simulink model "os_polyphase_synth_4096c"  (R2021a) (continued)
== system os_polyphase_synth_4096c/pfb_fir/pol1_in1_tap55 == (continued)
      | -P+(D+A)*BCIN+P
      | -P+(D+A)+C
      | -P+(D+A)+P
      | -P+(D+ACIN)
      | -P+(D+ACIN)*(D+ACIN)
      | -P+(D+ACIN)*(D+ACIN)+C
      | -P+(D+ACIN)*(D+ACIN)+P
      | -P+(D+ACIN)*B
      | -P+(D+ACIN)*B+C
      | -P+(D+ACIN)*B+P
      | -P+(D+ACIN)*BCIN
      | -P+(D+ACIN)*BCIN+C
      | -P+(D+ACIN)*BCIN+P
      | -P+(D+ACIN)+C
      | -P+(D+ACIN)+P
      | -P+(D-A)
      | -P+(D-A)*(D-A)
      | -P+(D-A)*(D-A)+C
      | -P+(D-A)*(D-A)+P
      | -P+(D-A)*B
      | -P+(D-A)*B+C
      | -P+(D-A)*B+P
      | -P+(D-A)*BCIN
      | -P+(D-A)*BCIN+C
      | -P+(D-A)*BCIN+P
      | -P+(D-A)+C
      | -P+(D-A)+P
      | -P+(D-ACIN)
      | -P+(D-ACIN)*(D-ACIN)
      | -P+(D-ACIN)*(D-ACIN)+C
      | -P+(D-ACIN)*(D-ACIN)+P
      | -P+(D-ACIN)*B
      | -P+(D-ACIN)*B+C
      | -P+(D-ACIN)*B+P
      | -P+(D-ACIN)*BCIN
      | -P+(D-ACIN)*BCIN+C
      | -P+(D-ACIN)*BCIN+P
      | -P+(D-ACIN)+C
      | -P+(D-ACIN)+P
      | -P+A
      | -P+A*A
      | -P+A*A+C
      | -P+A*A+P
      | -P+A*B
      | -P+A*B+C
      | -P+A*B+P
      | -P+A*BCIN
      | -P+A*BCIN+C
      | -P+A*BCIN+P
      | -P+A+C
      | -P+A+P
      | -P+ACIN
      | -P+ACIN*ACIN
      | -P+ACIN*ACIN+C
      | -P+ACIN*ACIN+P
      | -P+ACIN*B
      | -P+ACIN*B+C
      | -P+ACIN*B+P
      | -P+ACIN*BCIN
      | -P+ACIN*BCIN+C
      | -P+ACIN*BCIN+P
      | -P+ACIN+C
      | -P+ACIN+P
      | -P+B*(A+D)
      | -P+B*(A+D)+C
      | -P+B*(A+D)+P
      | -P+B*(ACIN+D)
      | -P+B*(ACIN+D)+C
      | -P+B*(ACIN+D)+P
      | -P+B*(D+A)
      | -P+B*(D+A)+C
      | -P+B*(D+A)+P
      | -P+B*(D+ACIN)
      | -P+B*(D+ACIN)+C
      | -P+B*(D+ACIN)+P
      | -P+B*(D-A)
      | -P+B*(D-A)+C
      | -P+B*(D-A)+P
      | -P+B*(D-ACIN)
      | -P+B*(D-ACIN)+C
      | -P+B*(D-ACIN)+P
      | -P+B*A
      | -P+B*A+C
      | -P+B*A+P
      | -P+B*ACIN
      | -P+B*ACIN+C
      | -P+B*ACIN+P
      | -P+B*D
      | -P+B*D+C
      | -P+B*D+P
      | -P+BCIN*(A+D)
      | -P+BCIN*(A+D)+C
      | -P+BCIN*(A+D)+P
      | -P+BCIN*(ACIN+D)
      | -P+BCIN*(ACIN+D)+C
      | -P+BCIN*(ACIN+D)+P
      | -P+BCIN*(D+A)
      | -P+BCIN*(D+A)+C
      | -P+BCIN*(D+A)+P
      | -P+BCIN*(D+ACIN)
      | -P+BCIN*(D+ACIN)+C
      | -P+BCIN*(D+ACIN)+P
      | -P+BCIN*(D-A)
      | -P+BCIN*(D-A)+C
      | -P+BCIN*(D-A)+P
      | -P+BCIN*(D-ACIN)
      | -P+BCIN*(D-ACIN)+C
      | -P+BCIN*(D-ACIN)+P
      | -P+BCIN*A
      | -P+BCIN*A+C
      | -P+BCIN*A+P
      | -P+BCIN*ACIN
      | -P+BCIN*ACIN+C
      | -P+BCIN*ACIN+P
      | -P+BCIN*D
      | -P+BCIN*D+C
      | -P+BCIN*D+P
      | -P+C
      | -P+C+(A+D)
      | -P+C+(A+D)*(A+D)
      | -P+C+(A+D)*B
      | -P+C+(A+D)*BCIN
      | -P+C+(ACIN+D)
      | -P+C+(ACIN+D)*(ACIN+D)
      | -P+C+(ACIN+D)*B
      | -P+C+(ACIN+D)*BCIN
      | -P+C+(D+A)
      | -P+C+(D+A)*(D+A)
      | -P+C+(D+A)*B
      | -P+C+(D+A)*BCIN
      | -P+C+(D+ACIN)
      | -P+C+(D+ACIN)*(D+ACIN)
      | -P+C+(D+ACIN)*B
      | -P+C+(D+ACIN)*BCIN
      | -P+C+(D-A)
      | -P+C+(D-A)*(D-A)
      | -P+C+(D-A)*B
      | -P+C+(D-A)*BCIN
      | -P+C+(D-ACIN)
      | -P+C+(D-ACIN)*(D-ACIN)
      | -P+C+(D-ACIN)*B
      | -P+C+(D-ACIN)*BCIN
      | -P+C+A
      | -P+C+A*A
      | -P+C+A*B
      | -P+C+A*BCIN
      | -P+C+ACIN
      | -P+C+ACIN*ACIN
      | -P+C+ACIN*B
      | -P+C+ACIN*BCIN
      | -P+C+B*(A+D)
      | -P+C+B*(ACIN+D)
      | -P+C+B*(D+A)
      | -P+C+B*(D+ACIN)
      | -P+C+B*(D-A)
      | -P+C+B*(D-ACIN)
      | -P+C+B*A
      | -P+C+B*ACIN
      | -P+C+B*D
      | -P+C+BCIN*(A+D)
      | -P+C+BCIN*(ACIN+D)
      | -P+C+BCIN*(D+A)
      | -P+C+BCIN*(D+ACIN)
      | -P+C+BCIN*(D-A)
      | -P+C+BCIN*(D-ACIN)
      | -P+C+BCIN*A
      | -P+C+BCIN*ACIN
      | -P+C+BCIN*D
      | -P+C+C
      | -P+C+C+CONCAT
      | -P+C+C+P
      | -P+C+CONCAT
      | -P+C+CONCAT+C
      | -P+C+CONCAT+P
      | -P+C+D
      | -P+C+D*B
      | -P+C+D*BCIN
      | -P+C+D*D
      | -P+C+P
      | -P+C+P+C
      | -P+C+P+CONCAT
      | -P+C+P+P
      | -P+CONCAT
      | -P+CONCAT+C
      | -P+CONCAT+C+C
      | -P+CONCAT+C+P
      | -P+CONCAT+P
      | -P+CONCAT+P+C
      | -P+D
      | -P+D*B
      | -P+D*B+C
      | -P+D*B+P
      | -P+D*BCIN
      | -P+D*BCIN+C
      | -P+D*BCIN+P
      | -P+D*D
      | -P+D*D+C
      | -P+D*D+P
      | -P+D+C
      | -P+D+P
      | -P+P
      | -P+P+(A+D)
      | -P+P+(A+D)*(A+D)
      | -P+P+(A+D)*B
      | -P+P+(A+D)*BCIN
      | -P+P+(ACIN+D)
      | -P+P+(ACIN+D)*(ACIN+D)
      | -P+P+(ACIN+D)*B
      | -P+P+(ACIN+D)*BCIN
      | -P+P+(D+A)
      | -P+P+(D+A)*(D+A)
      | -P+P+(D+A)*B
      | -P+P+(D+A)*BCIN
      | -P+P+(D+ACIN)
      | -P+P+(D+ACIN)*(D+ACIN)
      | -P+P+(D+ACIN)*B
      | -P+P+(D+ACIN)*BCIN
      | -P+P+(D-A)
      | -P+P+(D-A)*(D-A)
      | -P+P+(D-A)*B
      | -P+P+(D-A)*BCIN
      | -P+P+(D-ACIN)
      | -P+P+(D-ACIN)*(D-ACIN)
      | -P+P+(D-ACIN)*B
      | -P+P+(D-ACIN)*BCIN
      | -P+P+A
      | -P+P+A*A
      | -P+P+A*B
      | -P+P+A*BCIN
      | -P+P+ACIN
      | -P+P+ACIN*ACIN
      | -P+P+ACIN*B
      | -P+P+ACIN*BCIN
      | -P+P+B*(A+D)
      | -P+P+B*(ACIN+D)
      | -P+P+B*(D+A)
      | -P+P+B*(D+ACIN)
      | -P+P+B*(D-A)
      | -P+P+B*(D-ACIN)
      | -P+P+B*A
      | -P+P+B*ACIN
      | -P+P+B*D
      | -P+P+BCIN*(A+D)
      | -P+P+BCIN*(ACIN+D)
      | -P+P+BCIN*(D+A)
      | -P+P+BCIN*(D+ACIN)
      | -P+P+BCIN*(D-A)
      | -P+P+BCIN*(D-ACIN)
      | -P+P+BCIN*A
      | -P+P+BCIN*ACIN
      | -P+P+BCIN*D
      | -P+P+C
      | -P+P+C+C
      | -P+P+C+CONCAT
      | -P+P+C+P
      | -P+P+CONCAT
      | -P+P+CONCAT+C
      | -P+P+D
      | -P+P+D*B
      | -P+P+D*BCIN
      | -P+P+D*D
      | -P+P+P
      | -P+P+P+C
      | -P-((A+D))
      | -P-((A+D)*(A+D))
      | -P-((A+D)*B)
      | -P-((A+D)*BCIN)
      | -P-((ACIN+D))
      | -P-((ACIN+D)*(ACIN+D))
      | -P-((ACIN+D)*B)
      | -P-((ACIN+D)*BCIN)
      | -P-((D+A))
      | -P-((D+A)*(D+A))
      | -P-((D+A)*B)
      | -P-((D+A)*BCIN)
      | -P-((D+ACIN))
      | -P-((D+ACIN)*(D+ACIN))
      | -P-((D+ACIN)*B)
      | -P-((D+ACIN)*BCIN)
      | -P-((D-A))
      | -P-((D-A)*(D-A))
      | -P-((D-A)*B)
      | -P-((D-A)*BCIN)
      | -P-((D-ACIN))
      | -P-((D-ACIN)*(D-ACIN))
      | -P-((D-ACIN)*B)
      | -P-((D-ACIN)*BCIN)
      | -P-(A)
      | -P-(A*A)
      | -P-(A*B)
      | -P-(A*BCIN)
      | -P-(A+D)
      | -P-(A+D)*(A+D)
      | -P-(A+D)*(A+D)-CARRYIN
      | -P-(A+D)*B
      | -P-(A+D)*B-CARRYIN
      | -P-(A+D)*BCIN
      | -P-(A+D)*BCIN-CARRYIN
      | -P-(A+D)-CARRYIN
      | -P-(ACIN)
      | -P-(ACIN*ACIN)
      | -P-(ACIN*B)
      | -P-(ACIN*BCIN)
      | -P-(ACIN+D)
      | -P-(ACIN+D)*(ACIN+D)
      | -P-(ACIN+D)*(ACIN+D)-CARRYIN
      | -P-(ACIN+D)*B
      | -P-(ACIN+D)*B-CARRYIN
      | -P-(ACIN+D)*BCIN
      | -P-(ACIN+D)*BCIN-CARRYIN
      | -P-(ACIN+D)-CARRYIN
      | -P-(B*(A+D))
      | -P-(B*(ACIN+D))
      | -P-(B*(D+A))
      | -P-(B*(D+ACIN))
      | -P-(B*(D-A))
      | -P-(B*(D-ACIN))
      | -P-(B*A)
      | -P-(B*ACIN)
      | -P-(B*D)
      | -P-(BCIN*(A+D))
      | -P-(BCIN*(ACIN+D))
      | -P-(BCIN*(D+A))
      | -P-(BCIN*(D+ACIN))
      | -P-(BCIN*(D-A))
      | -P-(BCIN*(D-ACIN))
      | -P-(BCIN*A)
      | -P-(BCIN*ACIN)
      | -P-(BCIN*D)
      | -P-(D)
      | -P-(D*B)
      | -P-(D*BCIN)
      | -P-(D*D)
      | -P-(D+A)
      | -P-(D+A)*(D+A)
      | -P-(D+A)*(D+A)-CARRYIN
      | -P-(D+A)*B
      | -P-(D+A)*B-CARRYIN
      | -P-(D+A)*BCIN
      | -P-(D+A)*BCIN-CARRYIN
      | -P-(D+A)-CARRYIN
      | -P-(D+ACIN)
      | -P-(D+ACIN)*(D+ACIN)
      | -P-(D+ACIN)*(D+ACIN)-CARRYIN
      | -P-(D+ACIN)*B
      | -P-(D+ACIN)*B-CARRYIN
      | -P-(D+ACIN)*BCIN
      | -P-(D+ACIN)*BCIN-CARRYIN
      | -P-(D+ACIN)-CARRYIN
      | -P-(D-A)
      | -P-(D-A)*(D-A)
      | -P-(D-A)*(D-A)-CARRYIN
      | -P-(D-A)*B
      | -P-(D-A)*B-CARRYIN
      | -P-(D-A)*BCIN
      | -P-(D-A)*BCIN-CARRYIN
      | -P-(D-A)-CARRYIN
      | -P-(D-ACIN)
      | -P-(D-ACIN)*(D-ACIN)
      | -P-(D-ACIN)*(D-ACIN)-CARRYIN
      | -P-(D-ACIN)*B
      | -P-(D-ACIN)*B-CARRYIN
      | -P-(D-ACIN)*BCIN
      | -P-(D-ACIN)*BCIN-CARRYIN
      | -P-(D-ACIN)-CARRYIN
      | -P-A
      | -P-A*A
      | -P-A*A-CARRYIN
      | -P-A*B
      | -P-A*B-CARRYIN
      | -P-A*BCIN
      | -P-A*BCIN-CARRYIN
      | -P-A-CARRYIN
      | -P-ACIN
      | -P-ACIN*ACIN
      | -P-ACIN*ACIN-CARRYIN
      | -P-ACIN*B
      | -P-ACIN*B-CARRYIN
      | -P-ACIN*BCIN
      | -P-ACIN*BCIN-CARRYIN
      | -P-ACIN-CARRYIN
      | -P-B*(A+D)
      | -P-B*(A+D)-CARRYIN
      | -P-B*(ACIN+D)
      | -P-B*(ACIN+D)-CARRYIN
      | -P-B*(D+A)
      | -P-B*(D+A)-CARRYIN
      | -P-B*(D+ACIN)
      | -P-B*(D+ACIN)-CARRYIN
      | -P-B*(D-A)
      | -P-B*(D-A)-CARRYIN
      | -P-B*(D-ACIN)
      | -P-B*(D-ACIN)-CARRYIN
      | -P-B*A
      | -P-B*A-CARRYIN
      | -P-B*ACIN
      | -P-B*ACIN-CARRYIN
      | -P-B*D
      | -P-B*D-CARRYIN
      | -P-BCIN*(A+D)
      | -P-BCIN*(A+D)-CARRYIN
      | -P-BCIN*(ACIN+D)
      | -P-BCIN*(ACIN+D)-CARRYIN
      | -P-BCIN*(D+A)
      | -P-BCIN*(D+A)-CARRYIN
      | -P-BCIN*(D+ACIN)
      | -P-BCIN*(D+ACIN)-CARRYIN
      | -P-BCIN*(D-A)
      | -P-BCIN*(D-A)-CARRYIN
      | -P-BCIN*(D-ACIN)
      | -P-BCIN*(D-ACIN)-CARRYIN
      | -P-BCIN*A
      | -P-BCIN*A-CARRYIN
      | -P-BCIN*ACIN
      | -P-BCIN*ACIN-CARRYIN
      | -P-BCIN*D
      | -P-BCIN*D-CARRYIN
      | -P-C
      | -P-C-C
      | -P-C-C-CARRYIN
      | -P-C-CARRYCASCIN
      | -P-C-CARRYIN
      | -P-C-CONCAT
      | -P-C-CONCAT-CARRYIN
      | -P-C-P
      | -P-C-P-CARRYIN
      | -P-C-P>>17
      | -P-C-PCIN
      | -P-C-PCIN>>17
      | -P-CARRYCASCIN
      | -P-CARRYIN
      | -P-CONCAT
      | -P-CONCAT-C
      | -P-CONCAT-C-CARRYIN
      | -P-CONCAT-CARRYCASCIN
      | -P-CONCAT-CARRYIN
      | -P-CONCAT-P
      | -P-CONCAT-P>>17
      | -P-CONCAT-PCIN
      | -P-CONCAT-PCIN>>17
      | -P-D
      | -P-D*B
      | -P-D*B-CARRYIN
      | -P-D*BCIN
      | -P-D*BCIN-CARRYIN
      | -P-D*D
      | -P-D*D-CARRYIN
      | -P-D-CARRYIN
      | -P-P
      | -P-P-C
      | -P-P-C-CARRYIN
      | -P-P-CARRYCASCIN
      | -P-P-CARRYIN
      | -P-P-P
      | -P-P-P>>17
      | -P-P-PCIN
      | -P-P-PCIN>>17
      | -P-P>>17
      | -P-PCIN
      | -P-PCIN>>17
      | -P>>17
      | -P>>17+(A+D)
      | -P>>17+(A+D)*(A+D)
      | -P>>17+(A+D)*(A+D)+C
      | -P>>17+(A+D)*(A+D)+P
      | -P>>17+(A+D)*B
      | -P>>17+(A+D)*B+C
      | -P>>17+(A+D)*B+P
      | -P>>17+(A+D)*BCIN
      | -P>>17+(A+D)*BCIN+C
      | -P>>17+(A+D)*BCIN+P
      | -P>>17+(A+D)+C
      | -P>>17+(A+D)+P
      | -P>>17+(ACIN+D)
      | -P>>17+(ACIN+D)*(ACIN+D)
      | -P>>17+(ACIN+D)*(ACIN+D)+C
      | -P>>17+(ACIN+D)*(ACIN+D)+P
      | -P>>17+(ACIN+D)*B
      | -P>>17+(ACIN+D)*B+C
      | -P>>17+(ACIN+D)*B+P
      | -P>>17+(ACIN+D)*BCIN
      | -P>>17+(ACIN+D)*BCIN+C
      | -P>>17+(ACIN+D)*BCIN+P
      | -P>>17+(ACIN+D)+C
      | -P>>17+(ACIN+D)+P
      | -P>>17+(D+A)
      | -P>>17+(D+A)*(D+A)
      | -P>>17+(D+A)*(D+A)+C
      | -P>>17+(D+A)*(D+A)+P
      | -P>>17+(D+A)*B
      | -P>>17+(D+A)*B+C
      | -P>>17+(D+A)*B+P
      | -P>>17+(D+A)*BCIN
      | -P>>17+(D+A)*BCIN+C
      | -P>>17+(D+A)*BCIN+P
      | -P>>17+(D+A)+C
      | -P>>17+(D+A)+P
      | -P>>17+(D+ACIN)
      | -P>>17+(D+ACIN)*(D+ACIN)
      | -P>>17+(D+ACIN)*(D+ACIN)+C
      | -P>>17+(D+ACIN)*(D+ACIN)+P
      | -P>>17+(D+ACIN)*B
      | -P>>17+(D+ACIN)*B+C
      | -P>>17+(D+ACIN)*B+P
      | -P>>17+(D+ACIN)*BCIN
      | -P>>17+(D+ACIN)*BCIN+C
      | -P>>17+(D+ACIN)*BCIN+P
      | -P>>17+(D+ACIN)+C
      | -P>>17+(D+ACIN)+P
      | -P>>17+(D-A)
      | -P>>17+(D-A)*(D-A)
      | -P>>17+(D-A)*(D-A)+C
      | -P>>17+(D-A)*(D-A)+P
      | -P>>17+(D-A)*B
      | -P>>17+(D-A)*B+C
      | -P>>17+(D-A)*B+P
      | -P>>17+(D-A)*BCIN
      | -P>>17+(D-A)*BCIN+C
      | -P>>17+(D-A)*BCIN+P
      | -P>>17+(D-A)+C
      | -P>>17+(D-A)+P
      | -P>>17+(D-ACIN)
      | -P>>17+(D-ACIN)*(D-ACIN)
      | -P>>17+(D-ACIN)*(D-ACIN)+C
      | -P>>17+(D-ACIN)*(D-ACIN)+P
      | -P>>17+(D-ACIN)*B
      | -P>>17+(D-ACIN)*B+C
      | -P>>17+(D-ACIN)*B+P
      | -P>>17+(D-ACIN)*BCIN
      | -P>>17+(D-ACIN)*BCIN+C
      | -P>>17+(D-ACIN)*BCIN+P
      | -P>>17+(D-ACIN)+C
      | -P>>17+(D-ACIN)+P
      | -P>>17+A
      | -P>>17+A*A
      | -P>>17+A*A+C
      | -P>>17+A*A+P
      | -P>>17+A*B
      | -P>>17+A*B+C
      | -P>>17+A*B+P
      | -P>>17+A*BCIN
      | -P>>17+A*BCIN+C
      | -P>>17+A*BCIN+P
      | -P>>17+A+C
      | -P>>17+A+P
      | -P>>17+ACIN
      | -P>>17+ACIN*ACIN
      | -P>>17+ACIN*ACIN+C
      | -P>>17+ACIN*ACIN+P
      | -P>>17+ACIN*B
      | -P>>17+ACIN*B+C
      | -P>>17+ACIN*B+P
      | -P>>17+ACIN*BCIN
      | -P>>17+ACIN*BCIN+C
      | -P>>17+ACIN*BCIN+P
      | -P>>17+ACIN+C
      | -P>>17+ACIN+P
      | -P>>17+B*(A+D)
      | -P>>17+B*(A+D)+C
      | -P>>17+B*(A+D)+P
      | -P>>17+B*(ACIN+D)
      | -P>>17+B*(ACIN+D)+C
      | -P>>17+B*(ACIN+D)+P
      | -P>>17+B*(D+A)
      | -P>>17+B*(D+A)+C
      | -P>>17+B*(D+A)+P
      | -P>>17+B*(D+ACIN)
      | -P>>17+B*(D+ACIN)+C
      | -P>>17+B*(D+ACIN)+P
      | -P>>17+B*(D-A)
      | -P>>17+B*(D-A)+C
      | -P>>17+B*(D-A)+P
      | -P>>17+B*(D-ACIN)
      | -P>>17+B*(D-ACIN)+C
      | -P>>17+B*(D-ACIN)+P
      | -P>>17+B*A
      | -P>>17+B*A+C
      | -P>>17+B*A+P
      | -P>>17+B*ACIN
      | -P>>17+B*ACIN+C
      | -P>>17+B*ACIN+P
      | -P>>17+B*D
      | -P>>17+B*D+C
      | -P>>17+B*D+P
      | -P>>17+BCIN*(A+D)
      | -P>>17+BCIN*(A+D)+C
      | -P>>17+BCIN*(A+D)+P
      | -P>>17+BCIN*(ACIN+D)
      | -P>>17+BCIN*(ACIN+D)+C
      | -P>>17+BCIN*(ACIN+D)+P
      | -P>>17+BCIN*(D+A)
      | -P>>17+BCIN*(D+A)+C
      | -P>>17+BCIN*(D+A)+P
      | -P>>17+BCIN*(D+ACIN)
      | -P>>17+BCIN*(D+ACIN)+C
      | -P>>17+BCIN*(D+ACIN)+P
      | -P>>17+BCIN*(D-A)
      | -P>>17+BCIN*(D-A)+C
      | -P>>17+BCIN*(D-A)+P
      | -P>>17+BCIN*(D-ACIN)
      | -P>>17+BCIN*(D-ACIN)+C
      | -P>>17+BCIN*(D-ACIN)+P
      | -P>>17+BCIN*A
      | -P>>17+BCIN*A+C
      | -P>>17+BCIN*A+P
      | -P>>17+BCIN*ACIN
      | -P>>17+BCIN*ACIN+C
      | -P>>17+BCIN*ACIN+P
      | -P>>17+BCIN*D
      | -P>>17+BCIN*D+C
      | -P>>17+BCIN*D+P
      | -P>>17+C
      | -P>>17+C+(A+D)
      | -P>>17+C+(A+D)*(A+D)
      | -P>>17+C+(A+D)*B
      | -P>>17+C+(A+D)*BCIN
      | -P>>17+C+(ACIN+D)
      | -P>>17+C+(ACIN+D)*(ACIN+D)
      | -P>>17+C+(ACIN+D)*B
      | -P>>17+C+(ACIN+D)*BCIN
      | -P>>17+C+(D+A)
      | -P>>17+C+(D+A)*(D+A)
      | -P>>17+C+(D+A)*B
      | -P>>17+C+(D+A)*BCIN
      | -P>>17+C+(D+ACIN)
      | -P>>17+C+(D+ACIN)*(D+ACIN)
      | -P>>17+C+(D+ACIN)*B
      | -P>>17+C+(D+ACIN)*BCIN
      | -P>>17+C+(D-A)
      | -P>>17+C+(D-A)*(D-A)
      | -P>>17+C+(D-A)*B
      | -P>>17+C+(D-A)*BCIN
      | -P>>17+C+(D-ACIN)
      | -P>>17+C+(D-ACIN)*(D-ACIN)
      | -P>>17+C+(D-ACIN)*B
      | -P>>17+C+(D-ACIN)*BCIN
      | -P>>17+C+A
      | -P>>17+C+A*A
      | -P>>17+C+A*B
      | -P>>17+C+A*BCIN
      | -P>>17+C+ACIN
      | -P>>17+C+ACIN*ACIN
      | -P>>17+C+ACIN*B
      | -P>>17+C+ACIN*BCIN
      | -P>>17+C+B*(A+D)
      | -P>>17+C+B*(ACIN+D)
      | -P>>17+C+B*(D+A)
      | -P>>17+C+B*(D+ACIN)
      | -P>>17+C+B*(D-A)
      | -P>>17+C+B*(D-ACIN)
      | -P>>17+C+B*A
      | -P>>17+C+B*ACIN
      | -P>>17+C+B*D
      | -P>>17+C+BCIN*(A+D)
      | -P>>17+C+BCIN*(ACIN+D)
      | -P>>17+C+BCIN*(D+A)
      | -P>>17+C+BCIN*(D+ACIN)
      | -P>>17+C+BCIN*(D-A)
      | -P>>17+C+BCIN*(D-ACIN)
      | -P>>17+C+BCIN*A
      | -P>>17+C+BCIN*ACIN
      | -P>>17+C+BCIN*D
      | -P>>17+C+C
      | -P>>17+C+C+CONCAT
      | -P>>17+C+C+P
      | -P>>17+C+CONCAT
      | -P>>17+C+CONCAT+C
      | -P>>17+C+CONCAT+P
      | -P>>17+C+D
      | -P>>17+C+D*B
      | -P>>17+C+D*BCIN
      | -P>>17+C+D*D
      | -P>>17+C+P
      | -P>>17+C+P+C
      | -P>>17+C+P+CONCAT
      | -P>>17+C+P+P
      | -P>>17+CONCAT
      | -P>>17+CONCAT+C
      | -P>>17+CONCAT+C+C
      | -P>>17+CONCAT+C+P
      | -P>>17+CONCAT+P
      | -P>>17+CONCAT+P+C
      | -P>>17+D
      | -P>>17+D*B
      | -P>>17+D*B+C
      | -P>>17+D*B+P
      | -P>>17+D*BCIN
      | -P>>17+D*BCIN+C
      | -P>>17+D*BCIN+P
      | -P>>17+D*D
      | -P>>17+D*D+C
      | -P>>17+D*D+P
      | -P>>17+D+C
      | -P>>17+D+P
      | -P>>17+P
      | -P>>17+P+(A+D)
      | -P>>17+P+(A+D)*(A+D)
      | -P>>17+P+(A+D)*B
      | -P>>17+P+(A+D)*BCIN
      | -P>>17+P+(ACIN+D)
      | -P>>17+P+(ACIN+D)*(ACIN+D)
      | -P>>17+P+(ACIN+D)*B
      | -P>>17+P+(ACIN+D)*BCIN
      | -P>>17+P+(D+A)
      | -P>>17+P+(D+A)*(D+A)
      | -P>>17+P+(D+A)*B
      | -P>>17+P+(D+A)*BCIN
      | -P>>17+P+(D+ACIN)
      | -P>>17+P+(D+ACIN)*(D+ACIN)
      | -P>>17+P+(D+ACIN)*B
      | -P>>17+P+(D+ACIN)*BCIN
      | -P>>17+P+(D-A)
      | -P>>17+P+(D-A)*(D-A)
      | -P>>17+P+(D-A)*B
      | -P>>17+P+(D-A)*BCIN
      | -P>>17+P+(D-ACIN)
      | -P>>17+P+(D-ACIN)*(D-ACIN)
      | -P>>17+P+(D-ACIN)*B
      | -P>>17+P+(D-ACIN)*BCIN
      | -P>>17+P+A
      | -P>>17+P+A*A
      | -P>>17+P+A*B
      | -P>>17+P+A*BCIN
      | -P>>17+P+ACIN
      | -P>>17+P+ACIN*ACIN
      | -P>>17+P+ACIN*B
      | -P>>17+P+ACIN*BCIN
      | -P>>17+P+B*(A+D)
      | -P>>17+P+B*(ACIN+D)
      | -P>>17+P+B*(D+A)
      | -P>>17+P+B*(D+ACIN)
      | -P>>17+P+B*(D-A)
      | -P>>17+P+B*(D-ACIN)
      | -P>>17+P+B*A
      | -P>>17+P+B*ACIN
      | -P>>17+P+B*D
      | -P>>17+P+BCIN*(A+D)
      | -P>>17+P+BCIN*(ACIN+D)
      | -P>>17+P+BCIN*(D+A)
      | -P>>17+P+BCIN*(D+ACIN)
      | -P>>17+P+BCIN*(D-A)
      | -P>>17+P+BCIN*(D-ACIN)
      | -P>>17+P+BCIN*A
      | -P>>17+P+BCIN*ACIN
      | -P>>17+P+BCIN*D
      | -P>>17+P+C
      | -P>>17+P+C+C
      | -P>>17+P+C+CONCAT
      | -P>>17+P+C+P
      | -P>>17+P+CONCAT
      | -P>>17+P+CONCAT+C
      | -P>>17+P+D
      | -P>>17+P+D*B
      | -P>>17+P+D*BCIN
      | -P>>17+P+D*D
      | -P>>17+P+P
      | -P>>17+P+P+C
      | -P>>17-((A+D))
      | -P>>17-((A+D)*(A+D))
      | -P>>17-((A+D)*B)
      | -P>>17-((A+D)*BCIN)
      | -P>>17-((ACIN+D))
      | -P>>17-((ACIN+D)*(ACIN+D))
      | -P>>17-((ACIN+D)*B)
      | -P>>17-((ACIN+D)*BCIN)
      | -P>>17-((D+A))
      | -P>>17-((D+A)*(D+A))
      | -P>>17-((D+A)*B)
      | -P>>17-((D+A)*BCIN)
      | -P>>17-((D+ACIN))
      | -P>>17-((D+ACIN)*(D+ACIN))
      | -P>>17-((D+ACIN)*B)
      | -P>>17-((D+ACIN)*BCIN)
      | -P>>17-((D-A))
      | -P>>17-((D-A)*(D-A))
      | -P>>17-((D-A)*B)
      | -P>>17-((D-A)*BCIN)
      | -P>>17-((D-ACIN))
      | -P>>17-((D-ACIN)*(D-ACIN))
      | -P>>17-((D-ACIN)*B)
      | -P>>17-((D-ACIN)*BCIN)
      | -P>>17-(A)
      | -P>>17-(A*A)
      | -P>>17-(A*B)
      | -P>>17-(A*BCIN)
      | -P>>17-(ACIN)
      | -P>>17-(ACIN*ACIN)
      | -P>>17-(ACIN*B)
      | -P>>17-(ACIN*BCIN)
      | -P>>17-(B*(A+D))
      | -P>>17-(B*(ACIN+D))
      | -P>>17-(B*(D+A))
      | -P>>17-(B*(D+ACIN))
      | -P>>17-(B*(D-A))
      | -P>>17-(B*(D-ACIN))
      | -P>>17-(B*A)
      | -P>>17-(B*ACIN)
      | -P>>17-(B*D)
      | -P>>17-(BCIN*(A+D))
      | -P>>17-(BCIN*(ACIN+D))
      | -P>>17-(BCIN*(D+A))
      | -P>>17-(BCIN*(D+ACIN))
      | -P>>17-(BCIN*(D-A))
      | -P>>17-(BCIN*(D-ACIN))
      | -P>>17-(BCIN*A)
      | -P>>17-(BCIN*ACIN)
      | -P>>17-(BCIN*D)
      | -P>>17-(D)
      | -P>>17-(D*B)
      | -P>>17-(D*BCIN)
      | -P>>17-(D*D)
      | -PCIN
      | -PCIN+(A+D)
      | -PCIN+(A+D)*(A+D)
      | -PCIN+(A+D)*(A+D)+C
      | -PCIN+(A+D)*(A+D)+P
      | -PCIN+(A+D)*B
      | -PCIN+(A+D)*B+C
      | -PCIN+(A+D)*B+P
      | -PCIN+(A+D)*BCIN
      | -PCIN+(A+D)*BCIN+C
      | -PCIN+(A+D)*BCIN+P
      | -PCIN+(A+D)+C
      | -PCIN+(A+D)+P
      | -PCIN+(ACIN+D)
      | -PCIN+(ACIN+D)*(ACIN+D)
      | -PCIN+(ACIN+D)*(ACIN+D)+C
      | -PCIN+(ACIN+D)*(ACIN+D)+P
      | -PCIN+(ACIN+D)*B
      | -PCIN+(ACIN+D)*B+C
      | -PCIN+(ACIN+D)*B+P
      | -PCIN+(ACIN+D)*BCIN
      | -PCIN+(ACIN+D)*BCIN+C
      | -PCIN+(ACIN+D)*BCIN+P
      | -PCIN+(ACIN+D)+C
      | -PCIN+(ACIN+D)+P
      | -PCIN+(D+A)
      | -PCIN+(D+A)*(D+A)
      | -PCIN+(D+A)*(D+A)+C
      | -PCIN+(D+A)*(D+A)+P
      | -PCIN+(D+A)*B
      | -PCIN+(D+A)*B+C
      | -PCIN+(D+A)*B+P
      | -PCIN+(D+A)*BCIN
      | -PCIN+(D+A)*BCIN+C
      | -PCIN+(D+A)*BCIN+P
      | -PCIN+(D+A)+C
      | -PCIN+(D+A)+P
      | -PCIN+(D+ACIN)
      | -PCIN+(D+ACIN)*(D+ACIN)
      | -PCIN+(D+ACIN)*(D+ACIN)+C
      | -PCIN+(D+ACIN)*(D+ACIN)+P
      | -PCIN+(D+ACIN)*B
      | -PCIN+(D+ACIN)*B+C
      | -PCIN+(D+ACIN)*B+P
      | -PCIN+(D+ACIN)*BCIN
      | -PCIN+(D+ACIN)*BCIN+C
      | -PCIN+(D+ACIN)*BCIN+P
      | -PCIN+(D+ACIN)+C
      | -PCIN+(D+ACIN)+P
      | -PCIN+(D-A)
      | -PCIN+(D-A)*(D-A)
      | -PCIN+(D-A)*(D-A)+C
      | -PCIN+(D-A)*(D-A)+P
      | -PCIN+(D-A)*B
      | -PCIN+(D-A)*B+C
      | -PCIN+(D-A)*B+P
      | -PCIN+(D-A)*BCIN
      | -PCIN+(D-A)*BCIN+C
      | -PCIN+(D-A)*BCIN+P
      | -PCIN+(D-A)+C
      | -PCIN+(D-A)+P
      | -PCIN+(D-ACIN)
      | -PCIN+(D-ACIN)*(D-ACIN)
      | -PCIN+(D-ACIN)*(D-ACIN)+C
      | -PCIN+(D-ACIN)*(D-ACIN)+P
      | -PCIN+(D-ACIN)*B
      | -PCIN+(D-ACIN)*B+C
      | -PCIN+(D-ACIN)*B+P
      | -PCIN+(D-ACIN)*BCIN
      | -PCIN+(D-ACIN)*BCIN+C
      | -PCIN+(D-ACIN)*BCIN+P
      | -PCIN+(D-ACIN)+C
      | -PCIN+(D-ACIN)+P
      | -PCIN+A
      | -PCIN+A*A
      | -PCIN+A*A+C
      | -PCIN+A*A+P
      | -PCIN+A*B
      | -PCIN+A*B+C
      | -PCIN+A*B+P
      | -PCIN+A*BCIN
      | -PCIN+A*BCIN+C
      | -PCIN+A*BCIN+P
      | -PCIN+A+C
      | -PCIN+A+P
      | -PCIN+ACIN
      | -PCIN+ACIN*ACIN
      | -PCIN+ACIN*ACIN+C
      | -PCIN+ACIN*ACIN+P
      | -PCIN+ACIN*B
      | -PCIN+ACIN*B+C
      | -PCIN+ACIN*B+P
      | -PCIN+ACIN*BCIN
      | -PCIN+ACIN*BCIN+C
      | -PCIN+ACIN*BCIN+P
      | -PCIN+ACIN+C
      | -PCIN+ACIN+P
      | -PCIN+B*(A+D)
      | -PCIN+B*(A+D)+C
      | -PCIN+B*(A+D)+P
      | -PCIN+B*(ACIN+D)
      | -PCIN+B*(ACIN+D)+C
      | -PCIN+B*(ACIN+D)+P
      | -PCIN+B*(D+A)
      | -PCIN+B*(D+A)+C
      | -PCIN+B*(D+A)+P
      | -PCIN+B*(D+ACIN)
      | -PCIN+B*(D+ACIN)+C
      | -PCIN+B*(D+ACIN)+P
      | -PCIN+B*(D-A)
      | -PCIN+B*(D-A)+C
      | -PCIN+B*(D-A)+P
      | -PCIN+B*(D-ACIN)
      | -PCIN+B*(D-ACIN)+C
      | -PCIN+B*(D-ACIN)+P
      | -PCIN+B*A
      | -PCIN+B*A+C
      | -PCIN+B*A+P
      | -PCIN+B*ACIN
      | -PCIN+B*ACIN+C
      | -PCIN+B*ACIN+P
      | -PCIN+B*D
      | -PCIN+B*D+C
      | -PCIN+B*D+P
      | -PCIN+BCIN*(A+D)
      | -PCIN+BCIN*(A+D)+C
      | -PCIN+BCIN*(A+D)+P
      | -PCIN+BCIN*(ACIN+D)
      | -PCIN+BCIN*(ACIN+D)+C
      | -PCIN+BCIN*(ACIN+D)+P
      | -PCIN+BCIN*(D+A)
      | -PCIN+BCIN*(D+A)+C
      | -PCIN+BCIN*(D+A)+P
      | -PCIN+BCIN*(D+ACIN)
      | -PCIN+BCIN*(D+ACIN)+C
      | -PCIN+BCIN*(D+ACIN)+P
      | -PCIN+BCIN*(D-A)
      | -PCIN+BCIN*(D-A)+C
      | -PCIN+BCIN*(D-A)+P
      | -PCIN+BCIN*(D-ACIN)
      | -PCIN+BCIN*(D-ACIN)+C
      | -PCIN+BCIN*(D-ACIN)+P
      | -PCIN+BCIN*A
      | -PCIN+BCIN*A+C
      | -PCIN+BCIN*A+P
      | -PCIN+BCIN*ACIN
      | -PCIN+BCIN*ACIN+C
      | -PCIN+BCIN*ACIN+P
      | -PCIN+BCIN*D
      | -PCIN+BCIN*D+C
      | -PCIN+BCIN*D+P
      | -PCIN+C
      | -PCIN+C+(A+D)
      | -PCIN+C+(A+D)*(A+D)
      | -PCIN+C+(A+D)*B
      | -PCIN+C+(A+D)*BCIN
      | -PCIN+C+(ACIN+D)
      | -PCIN+C+(ACIN+D)*(ACIN+D)
      | -PCIN+C+(ACIN+D)*B
      | -PCIN+C+(ACIN+D)*BCIN
      | -PCIN+C+(D+A)
      | -PCIN+C+(D+A)*(D+A)
      | -PCIN+C+(D+A)*B
      | -PCIN+C+(D+A)*BCIN
      | -PCIN+C+(D+ACIN)
      | -PCIN+C+(D+ACIN)*(D+ACIN)
      | -PCIN+C+(D+ACIN)*B
      | -PCIN+C+(D+ACIN)*BCIN
      | -PCIN+C+(D-A)
      | -PCIN+C+(D-A)*(D-A)
      | -PCIN+C+(D-A)*B
      | -PCIN+C+(D-A)*BCIN
      | -PCIN+C+(D-ACIN)
      | -PCIN+C+(D-ACIN)*(D-ACIN)
      | -PCIN+C+(D-ACIN)*B
      | -PCIN+C+(D-ACIN)*BCIN
      | -PCIN+C+A
      | -PCIN+C+A*A
      | -PCIN+C+A*B
      | -PCIN+C+A*BCIN
      | -PCIN+C+ACIN
      | -PCIN+C+ACIN*ACIN
      | -PCIN+C+ACIN*B
      | -PCIN+C+ACIN*BCIN
      | -PCIN+C+B*(A+D)
      | -PCIN+C+B*(ACIN+D)
      | -PCIN+C+B*(D+A)
      | -PCIN+C+B*(D+ACIN)
      | -PCIN+C+B*(D-A)
      | -PCIN+C+B*(D-ACIN)
      | -PCIN+C+B*A
      | -PCIN+C+B*ACIN
      | -PCIN+C+B*D
      | -PCIN+C+BCIN*(A+D)
      | -PCIN+C+BCIN*(ACIN+D)
      | -PCIN+C+BCIN*(D+A)
      | -PCIN+C+BCIN*(D+ACIN)
      | -PCIN+C+BCIN*(D-A)
      | -PCIN+C+BCIN*(D-ACIN)
      | -PCIN+C+BCIN*A
      | -PCIN+C+BCIN*ACIN
      | -PCIN+C+BCIN*D
      | -PCIN+C+C
      | -PCIN+C+C+CONCAT
      | -PCIN+C+C+P
      | -PCIN+C+CONCAT
      | -PCIN+C+CONCAT+C
      | -PCIN+C+CONCAT+P
      | -PCIN+C+D
      | -PCIN+C+D*B
      | -PCIN+C+D*BCIN
      | -PCIN+C+D*D
      | -PCIN+C+P
      | -PCIN+C+P+C
      | -PCIN+C+P+CONCAT
      | -PCIN+C+P+P
      | -PCIN+CONCAT
      | -PCIN+CONCAT+C
      | -PCIN+CONCAT+C+C
      | -PCIN+CONCAT+C+P
      | -PCIN+CONCAT+P
      | -PCIN+CONCAT+P+C
      | -PCIN+D
      | -PCIN+D*B
      | -PCIN+D*B+C
      | -PCIN+D*B+P
      | -PCIN+D*BCIN
      | -PCIN+D*BCIN+C
      | -PCIN+D*BCIN+P
      | -PCIN+D*D
      | -PCIN+D*D+C
      | -PCIN+D*D+P
      | -PCIN+D+C
      | -PCIN+D+P
      | -PCIN+P
      | -PCIN+P+(A+D)
      | -PCIN+P+(A+D)*(A+D)
      | -PCIN+P+(A+D)*B
      | -PCIN+P+(A+D)*BCIN
      | -PCIN+P+(ACIN+D)
      | -PCIN+P+(ACIN+D)*(ACIN+D)
      | -PCIN+P+(ACIN+D)*B
      | -PCIN+P+(ACIN+D)*BCIN
      | -PCIN+P+(D+A)
      | -PCIN+P+(D+A)*(D+A)
      | -PCIN+P+(D+A)*B
      | -PCIN+P+(D+A)*BCIN
      | -PCIN+P+(D+ACIN)
      | -PCIN+P+(D+ACIN)*(D+ACIN)
      | -PCIN+P+(D+ACIN)*B
      | -PCIN+P+(D+ACIN)*BCIN
      | -PCIN+P+(D-A)
      | -PCIN+P+(D-A)*(D-A)
      | -PCIN+P+(D-A)*B
      | -PCIN+P+(D-A)*BCIN
      | -PCIN+P+(D-ACIN)
      | -PCIN+P+(D-ACIN)*(D-ACIN)
      | -PCIN+P+(D-ACIN)*B
      | -PCIN+P+(D-ACIN)*BCIN
      | -PCIN+P+A
      | -PCIN+P+A*A
      | -PCIN+P+A*B
      | -PCIN+P+A*BCIN
      | -PCIN+P+ACIN
      | -PCIN+P+ACIN*ACIN
      | -PCIN+P+ACIN*B
      | -PCIN+P+ACIN*BCIN
      | -PCIN+P+B*(A+D)
      | -PCIN+P+B*(ACIN+D)
      | -PCIN+P+B*(D+A)
      | -PCIN+P+B*(D+ACIN)
      | -PCIN+P+B*(D-A)
      | -PCIN+P+B*(D-ACIN)
      | -PCIN+P+B*A
      | -PCIN+P+B*ACIN
      | -PCIN+P+B*D
      | -PCIN+P+BCIN*(A+D)
      | -PCIN+P+BCIN*(ACIN+D)
      | -PCIN+P+BCIN*(D+A)
      | -PCIN+P+BCIN*(D+ACIN)
      | -PCIN+P+BCIN*(D-A)
      | -PCIN+P+BCIN*(D-ACIN)
      | -PCIN+P+BCIN*A
      | -PCIN+P+BCIN*ACIN
      | -PCIN+P+BCIN*D
      | -PCIN+P+C
      | -PCIN+P+C+C
      | -PCIN+P+C+CONCAT
      | -PCIN+P+C+P
      | -PCIN+P+CONCAT
      | -PCIN+P+CONCAT+C
      | -PCIN+P+D
      | -PCIN+P+D*B
      | -PCIN+P+D*BCIN
      | -PCIN+P+D*D
      | -PCIN+P+P
      | -PCIN+P+P+C
      | -PCIN-((A+D))
      | -PCIN-((A+D)*(A+D))
      | -PCIN-((A+D)*B)
      | -PCIN-((A+D)*BCIN)
      | -PCIN-((ACIN+D))
      | -PCIN-((ACIN+D)*(ACIN+D))
      | -PCIN-((ACIN+D)*B)
      | -PCIN-((ACIN+D)*BCIN)
      | -PCIN-((D+A))
      | -PCIN-((D+A)*(D+A))
      | -PCIN-((D+A)*B)
      | -PCIN-((D+A)*BCIN)
      | -PCIN-((D+ACIN))
      | -PCIN-((D+ACIN)*(D+ACIN))
      | -PCIN-((D+ACIN)*B)
      | -PCIN-((D+ACIN)*BCIN)
      | -PCIN-((D-A))
      | -PCIN-((D-A)*(D-A))
      | -PCIN-((D-A)*B)
      | -PCIN-((D-A)*BCIN)
      | -PCIN-((D-ACIN))
      | -PCIN-((D-ACIN)*(D-ACIN))
      | -PCIN-((D-ACIN)*B)
      | -PCIN-((D-ACIN)*BCIN)
      | -PCIN-(A)
      | -PCIN-(A*A)
      | -PCIN-(A*B)
      | -PCIN-(A*BCIN)
      | -PCIN-(ACIN)
      | -PCIN-(ACIN*ACIN)
      | -PCIN-(ACIN*B)
      | -PCIN-(ACIN*BCIN)
      | -PCIN-(B*(A+D))
      | -PCIN-(B*(ACIN+D))
      | -PCIN-(B*(D+A))
      | -PCIN-(B*(D+ACIN))
      | -PCIN-(B*(D-A))
      | -PCIN-(B*(D-ACIN))
      | -PCIN-(B*A)
      | -PCIN-(B*ACIN)
      | -PCIN-(B*D)
      | -PCIN-(BCIN*(A+D))
      | -PCIN-(BCIN*(ACIN+D))
      | -PCIN-(BCIN*(D+A))
      | -PCIN-(BCIN*(D+ACIN))
      | -PCIN-(BCIN*(D-A))
      | -PCIN-(BCIN*(D-ACIN))
      | -PCIN-(BCIN*A)
      | -PCIN-(BCIN*ACIN)
      | -PCIN-(BCIN*D)
      | -PCIN-(D)
      | -PCIN-(D*B)
      | -PCIN-(D*BCIN)
      | -PCIN-(D*D)
      | -PCIN>>17
      | -PCIN>>17+(A+D)
      | -PCIN>>17+(A+D)*(A+D)
      | -PCIN>>17+(A+D)*(A+D)+C
      | -PCIN>>17+(A+D)*(A+D)+P
      | -PCIN>>17+(A+D)*B
      | -PCIN>>17+(A+D)*B+C
      | -PCIN>>17+(A+D)*B+P
      | -PCIN>>17+(A+D)*BCIN
      | -PCIN>>17+(A+D)*BCIN+C
      | -PCIN>>17+(A+D)*BCIN+P
      | -PCIN>>17+(A+D)+C
      | -PCIN>>17+(A+D)+P
      | -PCIN>>17+(ACIN+D)
      | -PCIN>>17+(ACIN+D)*(ACIN+D)
      | -PCIN>>17+(ACIN+D)*(ACIN+D)+C
      | -PCIN>>17+(ACIN+D)*(ACIN+D)+P
      | -PCIN>>17+(ACIN+D)*B
      | -PCIN>>17+(ACIN+D)*B+C
      | -PCIN>>17+(ACIN+D)*B+P
      | -PCIN>>17+(ACIN+D)*BCIN
      | -PCIN>>17+(ACIN+D)*BCIN+C
      | -PCIN>>17+(ACIN+D)*BCIN+P
      | -PCIN>>17+(ACIN+D)+C
      | -PCIN>>17+(ACIN+D)+P
      | -PCIN>>17+(D+A)
      | -PCIN>>17+(D+A)*(D+A)
      | -PCIN>>17+(D+A)*(D+A)+C
      | -PCIN>>17+(D+A)*(D+A)+P
      | -PCIN>>17+(D+A)*B
      | -PCIN>>17+(D+A)*B+C
      | -PCIN>>17+(D+A)*B+P
      | -PCIN>>17+(D+A)*BCIN
      | -PCIN>>17+(D+A)*BCIN+C
      | -PCIN>>17+(D+A)*BCIN+P
      | -PCIN>>17+(D+A)+C
      | -PCIN>>17+(D+A)+P
      | -PCIN>>17+(D+ACIN)
      | -PCIN>>17+(D+ACIN)*(D+ACIN)
      | -PCIN>>17+(D+ACIN)*(D+ACIN)+C
      | -PCIN>>17+(D+ACIN)*(D+ACIN)+P
      | -PCIN>>17+(D+ACIN)*B
      | -PCIN>>17+(D+ACIN)*B+C
      | -PCIN>>17+(D+ACIN)*B+P
      | -PCIN>>17+(D+ACIN)*BCIN
      | -PCIN>>17+(D+ACIN)*BCIN+C
      | -PCIN>>17+(D+ACIN)*BCIN+P
      | -PCIN>>17+(D+ACIN)+C
      | -PCIN>>17+(D+ACIN)+P
      | -PCIN>>17+(D-A)
      | -PCIN>>17+(D-A)*(D-A)
      | -PCIN>>17+(D-A)*(D-A)+C
      | -PCIN>>17+(D-A)*(D-A)+P
      | -PCIN>>17+(D-A)*B
      | -PCIN>>17+(D-A)*B+C
      | -PCIN>>17+(D-A)*B+P
      | -PCIN>>17+(D-A)*BCIN
      | -PCIN>>17+(D-A)*BCIN+C
      | -PCIN>>17+(D-A)*BCIN+P
      | -PCIN>>17+(D-A)+C
      | -PCIN>>17+(D-A)+P
      | -PCIN>>17+(D-ACIN)
      | -PCIN>>17+(D-ACIN)*(D-ACIN)
      | -PCIN>>17+(D-ACIN)*(D-ACIN)+C
      | -PCIN>>17+(D-ACIN)*(D-ACIN)+P
      | -PCIN>>17+(D-ACIN)*B
      | -PCIN>>17+(D-ACIN)*B+C
      | -PCIN>>17+(D-ACIN)*B+P
      | -PCIN>>17+(D-ACIN)*BCIN
      | -PCIN>>17+(D-ACIN)*BCIN+C
      | -PCIN>>17+(D-ACIN)*BCIN+P
      | -PCIN>>17+(D-ACIN)+C
      | -PCIN>>17+(D-ACIN)+P
      | -PCIN>>17+A
      | -PCIN>>17+A*A
      | -PCIN>>17+A*A+C
      | -PCIN>>17+A*A+P
      | -PCIN>>17+A*B
      | -PCIN>>17+A*B+C
      | -PCIN>>17+A*B+P
      | -PCIN>>17+A*BCIN
      | -PCIN>>17+A*BCIN+C
      | -PCIN>>17+A*BCIN+P
      | -PCIN>>17+A+C
      | -PCIN>>17+A+P
      | -PCIN>>17+ACIN
      | -PCIN>>17+ACIN*ACIN
      | -PCIN>>17+ACIN*ACIN+C
      | -PCIN>>17+ACIN*ACIN+P
      | -PCIN>>17+ACIN*B
      | -PCIN>>17+ACIN*B+C
      | -PCIN>>17+ACIN*B+P
      | -PCIN>>17+ACIN*BCIN
      | -PCIN>>17+ACIN*BCIN+C
      | -PCIN>>17+ACIN*BCIN+P
      | -PCIN>>17+ACIN+C
      | -PCIN>>17+ACIN+P
      | -PCIN>>17+B*(A+D)
      | -PCIN>>17+B*(A+D)+C
      | -PCIN>>17+B*(A+D)+P
      | -PCIN>>17+B*(ACIN+D)
      | -PCIN>>17+B*(ACIN+D)+C
      | -PCIN>>17+B*(ACIN+D)+P
      | -PCIN>>17+B*(D+A)
      | -PCIN>>17+B*(D+A)+C
      | -PCIN>>17+B*(D+A)+P
      | -PCIN>>17+B*(D+ACIN)
      | -PCIN>>17+B*(D+ACIN)+C
      | -PCIN>>17+B*(D+ACIN)+P
      | -PCIN>>17+B*(D-A)
      | -PCIN>>17+B*(D-A)+C
      | -PCIN>>17+B*(D-A)+P
      | -PCIN>>17+B*(D-ACIN)
      | -PCIN>>17+B*(D-ACIN)+C
      | -PCIN>>17+B*(D-ACIN)+P
      | -PCIN>>17+B*A
      | -PCIN>>17+B*A+C
      | -PCIN>>17+B*A+P
      | -PCIN>>17+B*ACIN
      | -PCIN>>17+B*ACIN+C
      | -PCIN>>17+B*ACIN+P
      | -PCIN>>17+B*D
      | -PCIN>>17+B*D+C
      | -PCIN>>17+B*D+P
      | -PCIN>>17+BCIN*(A+D)
      | -PCIN>>17+BCIN*(A+D)+C
      | -PCIN>>17+BCIN*(A+D)+P
      | -PCIN>>17+BCIN*(ACIN+D)
      | -PCIN>>17+BCIN*(ACIN+D)+C
      | -PCIN>>17+BCIN*(ACIN+D)+P
      | -PCIN>>17+BCIN*(D+A)
      | -PCIN>>17+BCIN*(D+A)+C
      | -PCIN>>17+BCIN*(D+A)+P
      | -PCIN>>17+BCIN*(D+ACIN)
      | -PCIN>>17+BCIN*(D+ACIN)+C
      | -PCIN>>17+BCIN*(D+ACIN)+P
      | -PCIN>>17+BCIN*(D-A)
      | -PCIN>>17+BCIN*(D-A)+C
      | -PCIN>>17+BCIN*(D-A)+P
      | -PCIN>>17+BCIN*(D-ACIN)
      | -PCIN>>17+BCIN*(D-ACIN)+C
      | -PCIN>>17+BCIN*(D-ACIN)+P
      | -PCIN>>17+BCIN*A
      | -PCIN>>17+BCIN*A+C
      | -PCIN>>17+BCIN*A+P
      | -PCIN>>17+BCIN*ACIN
      | -PCIN>>17+BCIN*ACIN+C
      | -PCIN>>17+BCIN*ACIN+P
      | -PCIN>>17+BCIN*D
      | -PCIN>>17+BCIN*D+C
      | -PCIN>>17+BCIN*D+P
      | -PCIN>>17+C
      | -PCIN>>17+C+(A+D)
      | -PCIN>>17+C+(A+D)*(A+D)
      | -PCIN>>17+C+(A+D)*B
      | -PCIN>>17+C+(A+D)*BCIN
      | -PCIN>>17+C+(ACIN+D)
      | -PCIN>>17+C+(ACIN+D)*(ACIN+D)
      | -PCIN>>17+C+(ACIN+D)*B
      | -PCIN>>17+C+(ACIN+D)*BCIN
      | -PCIN>>17+C+(D+A)
      | -PCIN>>17+C+(D+A)*(D+A)
      | -PCIN>>17+C+(D+A)*B
      | -PCIN>>17+C+(D+A)*BCIN
      | -PCIN>>17+C+(D+ACIN)
      | -PCIN>>17+C+(D+ACIN)*(D+ACIN)
      | -PCIN>>17+C+(D+ACIN)*B
      | -PCIN>>17+C+(D+ACIN)*BCIN
      | -PCIN>>17+C+(D-A)
      | -PCIN>>17+C+(D-A)*(D-A)
      | -PCIN>>17+C+(D-A)*B
      | -PCIN>>17+C+(D-A)*BCIN
      | -PCIN>>17+C+(D-ACIN)
      | -PCIN>>17+C+(D-ACIN)*(D-ACIN)
      | -PCIN>>17+C+(D-ACIN)*B
      | -PCIN>>17+C+(D-ACIN)*BCIN
      | -PCIN>>17+C+A
      | -PCIN>>17+C+A*A
      | -PCIN>>17+C+A*B
      | -PCIN>>17+C+A*BCIN
      | -PCIN>>17+C+ACIN
      | -PCIN>>17+C+ACIN*ACIN
      | -PCIN>>17+C+ACIN*B
      | -PCIN>>17+C+ACIN*BCIN
      | -PCIN>>17+C+B*(A+D)
      | -PCIN>>17+C+B*(ACIN+D)
      | -PCIN>>17+C+B*(D+A)
      | -PCIN>>17+C+B*(D+ACIN)
      | -PCIN>>17+C+B*(D-A)
      | -PCIN>>17+C+B*(D-ACIN)
      | -PCIN>>17+C+B*A
      | -PCIN>>17+C+B*ACIN
      | -PCIN>>17+C+B*D
      | -PCIN>>17+C+BCIN*(A+D)
      | -PCIN>>17+C+BCIN*(ACIN+D)
      | -PCIN>>17+C+BCIN*(D+A)
      | -PCIN>>17+C+BCIN*(D+ACIN)
      | -PCIN>>17+C+BCIN*(D-A)
      | -PCIN>>17+C+BCIN*(D-ACIN)
      | -PCIN>>17+C+BCIN*A
      | -PCIN>>17+C+BCIN*ACIN
      | -PCIN>>17+C+BCIN*D
      | -PCIN>>17+C+C
      | -PCIN>>17+C+C+CONCAT
      | -PCIN>>17+C+C+P
      | -PCIN>>17+C+CONCAT
      | -PCIN>>17+C+CONCAT+C
      | -PCIN>>17+C+CONCAT+P
      | -PCIN>>17+C+D
      | -PCIN>>17+C+D*B
      | -PCIN>>17+C+D*BCIN
      | -PCIN>>17+C+D*D
      | -PCIN>>17+C+P
      | -PCIN>>17+C+P+C
      | -PCIN>>17+C+P+CONCAT
      | -PCIN>>17+C+P+P
      | -PCIN>>17+CONCAT
      | -PCIN>>17+CONCAT+C
      | -PCIN>>17+CONCAT+C+C
      | -PCIN>>17+CONCAT+C+P
      | -PCIN>>17+CONCAT+P
      | -PCIN>>17+CONCAT+P+C
      | -PCIN>>17+D
      | -PCIN>>17+D*B
      | -PCIN>>17+D*B+C
      | -PCIN>>17+D*B+P
      | -PCIN>>17+D*BCIN
      | -PCIN>>17+D*BCIN+C
      | -PCIN>>17+D*BCIN+P
      | -PCIN>>17+D*D
      | -PCIN>>17+D*D+C
      | -PCIN>>17+D*D+P
      | -PCIN>>17+D+C
      | -PCIN>>17+D+P
      | -PCIN>>17+P
      | -PCIN>>17+P+(A+D)
      | -PCIN>>17+P+(A+D)*(A+D)
      | -PCIN>>17+P+(A+D)*B
      | -PCIN>>17+P+(A+D)*BCIN
      | -PCIN>>17+P+(ACIN+D)
      | -PCIN>>17+P+(ACIN+D)*(ACIN+D)
      | -PCIN>>17+P+(ACIN+D)*B
      | -PCIN>>17+P+(ACIN+D)*BCIN
      | -PCIN>>17+P+(D+A)
      | -PCIN>>17+P+(D+A)*(D+A)
      | -PCIN>>17+P+(D+A)*B
      | -PCIN>>17+P+(D+A)*BCIN
      | -PCIN>>17+P+(D+ACIN)
      | -PCIN>>17+P+(D+ACIN)*(D+ACIN)
      | -PCIN>>17+P+(D+ACIN)*B
      | -PCIN>>17+P+(D+ACIN)*BCIN
      | -PCIN>>17+P+(D-A)
      | -PCIN>>17+P+(D-A)*(D-A)
      | -PCIN>>17+P+(D-A)*B
      | -PCIN>>17+P+(D-A)*BCIN
      | -PCIN>>17+P+(D-ACIN)
      | -PCIN>>17+P+(D-ACIN)*(D-ACIN)
      | -PCIN>>17+P+(D-ACIN)*B
      | -PCIN>>17+P+(D-ACIN)*BCIN
      | -PCIN>>17+P+A
      | -PCIN>>17+P+A*A
      | -PCIN>>17+P+A*B
      | -PCIN>>17+P+A*BCIN
      | -PCIN>>17+P+ACIN
      | -PCIN>>17+P+ACIN*ACIN
      | -PCIN>>17+P+ACIN*B
      | -PCIN>>17+P+ACIN*BCIN
      | -PCIN>>17+P+B*(A+D)
      | -PCIN>>17+P+B*(ACIN+D)
      | -PCIN>>17+P+B*(D+A)
      | -PCIN>>17+P+B*(D+ACIN)
      | -PCIN>>17+P+B*(D-A)
      | -PCIN>>17+P+B*(D-ACIN)
      | -PCIN>>17+P+B*A
      | -PCIN>>17+P+B*ACIN
      | -PCIN>>17+P+B*D
      | -PCIN>>17+P+BCIN*(A+D)
      | -PCIN>>17+P+BCIN*(ACIN+D)
      | -PCIN>>17+P+BCIN*(D+A)
      | -PCIN>>17+P+BCIN*(D+ACIN)
      | -PCIN>>17+P+BCIN*(D-A)
      | -PCIN>>17+P+BCIN*(D-ACIN)
      | -PCIN>>17+P+BCIN*A
      | -PCIN>>17+P+BCIN*ACIN
      | -PCIN>>17+P+BCIN*D
      | -PCIN>>17+P+C
      | -PCIN>>17+P+C+C
      | -PCIN>>17+P+C+CONCAT
      | -PCIN>>17+P+C+P
      | -PCIN>>17+P+CONCAT
      | -PCIN>>17+P+CONCAT+C
      | -PCIN>>17+P+D
      | -PCIN>>17+P+D*B
      | -PCIN>>17+P+D*BCIN
      | -PCIN>>17+P+D*D
      | -PCIN>>17+P+P
      | -PCIN>>17+P+P+C
      | -PCIN>>17-((A+D))
      | -PCIN>>17-((A+D)*(A+D))
      | -PCIN>>17-((A+D)*B)
      | -PCIN>>17-((A+D)*BCIN)
      | -PCIN>>17-((ACIN+D))
      | -PCIN>>17-((ACIN+D)*(ACIN+D))
      | -PCIN>>17-((ACIN+D)*B)
      | -PCIN>>17-((ACIN+D)*BCIN)
      | -PCIN>>17-((D+A))
      | -PCIN>>17-((D+A)*(D+A))
      | -PCIN>>17-((D+A)*B)
      | -PCIN>>17-((D+A)*BCIN)
      | -PCIN>>17-((D+ACIN))
      | -PCIN>>17-((D+ACIN)*(D+ACIN))
      | -PCIN>>17-((D+ACIN)*B)
      | -PCIN>>17-((D+ACIN)*BCIN)
      | -PCIN>>17-((D-A))
      | -PCIN>>17-((D-A)*(D-A))
      | -PCIN>>17-((D-A)*B)
      | -PCIN>>17-((D-A)*BCIN)
      | -PCIN>>17-((D-ACIN))
      | -PCIN>>17-((D-ACIN)*(D-ACIN))
      | -PCIN>>17-((D-ACIN)*B)
      | -PCIN>>17-((D-ACIN)*BCIN)
      | -PCIN>>17-(A)
      | -PCIN>>17-(A*A)
      | -PCIN>>17-(A*B)
      | -PCIN>>17-(A*BCIN)
      | -PCIN>>17-(ACIN)
      | -PCIN>>17-(ACIN*ACIN)
      | -PCIN>>17-(ACIN*B)
      | -PCIN>>17-(ACIN*BCIN)
      | -PCIN>>17-(B*(A+D))
      | -PCIN>>17-(B*(ACIN+D))
      | -PCIN>>17-(B*(D+A))
      | -PCIN>>17-(B*(D+ACIN))
      | -PCIN>>17-(B*(D-A))
      | -PCIN>>17-(B*(D-ACIN))
      | -PCIN>>17-(B*A)
      | -PCIN>>17-(B*ACIN)
      | -PCIN>>17-(B*D)
      | -PCIN>>17-(BCIN*(A+D))
      | -PCIN>>17-(BCIN*(ACIN+D))
      | -PCIN>>17-(BCIN*(D+A))
      | -PCIN>>17-(BCIN*(D+ACIN))
      | -PCIN>>17-(BCIN*(D-A))
      | -PCIN>>17-(BCIN*(D-ACIN))
      | -PCIN>>17-(BCIN*A)
      | -PCIN>>17-(BCIN*ACIN)
      | -PCIN>>17-(BCIN*D)
      | -PCIN>>17-(D)
      | -PCIN>>17-(D*B)
      | -PCIN>>17-(D*BCIN)
      | -PCIN>>17-(D*D)